simulink model "TosAeb"  (R2021a)
settings: Solver=FixedStepDiscrete  StartTime=0.0  StopTime=inf  FixedStep=0.01  RelTol=1e-3
undefined symbols (not defined in the file or in its folder): ACCModSts, AccSts1, AEB_ObjectMovingOrientation, AEB_ObjectMovingState, CN_BTCActive, CN_CurrentGearR, CN_CurrentGearR_DHT, CN_PTCActive, CN_VDCActive, CN_VSC_EspOff, CN_VSC_FCW_AEB_Error, CN_VSC_FCW_AEB_NoDispay, CN_VSC_FCW_AEB_PerfmDgdtn, CN_VSC_FCW_AEB_TempUnAvlbl, CN_VSC_VehStandstill, CNaccelAvoidMax, CNaccelAvoidMin, CNbrakePedalPress, CNcomfortStrLeftAccelMax, CNcomfortStrLeftAccelMin, CNcomfortStrRightAccelMax, CNcomfortStrRightAccelMin, CNDrvDoorOpen, CNDrvModeEco, CNDrvModeNorm, CNDrvModeOther, CNDrvModeSport, CNDrvSBRreminder, CNNull, CNobjInvalidID, CNPassDoorOpen, CNPassSBRreminder, CNPEBSAccelNegInf, CNPEBSAccelNull, CNPEBSTimeInf, CNPEBSTimeNull, CNSTOPMAX, CNSTOPNULL, CNsubjWidth, CNSwtOFF, CNSwtON, CNTTCMAX, CNTTCNULL, CNVehConfigAEBCycNone, CNVehConfigAEBNone, CNVehConfigAEBPedNone, CNVehConfigFCWNone, CNVehConfigJANone, CollisionSeverity, FALSE, FCWSnvty, JACycObjCalcInfoDefaultParams, JAPedObjCalcInfoDefaultParams, JAVehObjCalcInfoDefaultParams, latCycObjCalcInfoDefaultParams, latPedObjCalcInfoDefaultParams, longObjCalcInfoDefaultParams, ModSts, movingStatus, ObjectType ... (+1112)
libraries: simulink x3031, AEBSFunctionLib x50, Ta_Library x33, AEBSStateMachineLib x23, Mylibrary x12, simulink_extras x7, ObjSelect_lib x3, dspstat3 x2
(part 53 of 102)

== system TosAeb/FcwAebJa_Step_sys/ALG_AEBSystem/ALG_AEBController/AEB_FuncCondCalcApp/LongFunctionCondCalc/vehEBASuppAborCondCalc/vehEBASuppCondCalc/vehEBASituationRelActiveCondCalc/ESPmasterCylindBrakePressDt ==
  ESPmasterCylindBrakePress : Inport
  Constant : Constant  Value=1/Ts
  Gain : Gain  Gain=1/4
  Product : Product
  Subtract : Sum  Inputs=+-
  Sum : Sum  Inputs=+
  Tapped Delay : simulink/Discrete/Tapped Delay  vinit=0.0  samptime=-1  NumDelays=3  DelayOrder=Newest  includeCurrent=on
  Unit Delay : UnitDelay  SampleTime=-1  HasFrameUpgradeWarning=on
  ESPmasterCylindBrakePressDt : Outport
  wires:
  Sum/1 -> Gain/1
  Unit Delay/1 -> Subtract/2
  ESPmasterCylindBrakePress/1 -> Unit Delay/1, Subtract/1
  Subtract/1 -> Product/1
  Constant/1 -> Product/2
  Gain/1 -> ESPmasterCylindBrakePressDt/1
  Product/1 -> Tapped Delay/1
  Tapped Delay/1 -> Sum/1

== system TosAeb/FcwAebJa_Step_sys/ALG_AEBSystem/ALG_AEBController/AEB_FuncCondCalcApp/LongFunctionCondCalc/vehEBASuppAborCondCalc/vehEBASuppCondCalc/vehEBASituationRelActiveCondCalc/brakeReleventSuppCond ==
  VehConfigIbooster : Inport
  predictiveAccelAvoid : Inport  Port=2
  ObjectMovingState : Inport  Port=3
  subjVel : Inport  Port=4
  ESPmasterCylindBrakePress : Inport  Port=5
  BrakePedalStatus_G : Inport  Port=6
  subjLongAccelFromSensor : Inport  Port=7
  subjLongAccel : Inport  Port=8
  IB_BrkPedalAppld : Inport  Port=9
  Bus Creator : BusCreator  Inputs=8
  Bus Selector : BusSelector  OutputSignals=predictiveAccelAvoid,ObjectMovingState,subjVel,ESPmasterCylindBrakePress,BrakePedalStatus_G,subjLongAccelFromSensor,subjLongAccel
  Bus Selector1 : BusSelector  OutputSignals=predictiveAccelAvoid,ObjectMovingState,subjVel,subjLongAccelFromSensor,subjLongAccel,IB_BrkPedalAppld
  Compare To Constant : simulink/Logic and Bit Operations/Compare To Constant  relop===  const=FALSE  OutDataTypeStr=boolean
  If : If  IfExpression=u1
  Merge : Merge
  brakeReleventSuppCond_ESP : Subsystem  VariantControl=(default)
  brakeReleventSuppCond_IBooster : Subsystem  VariantControl=P_AEB_PowerTrainConfig == 1
  brakeReleventSuppCond : Outport
  wires:
  If/2 -> brakeReleventSuppCond_IBooster/Action
  Bus Selector1/1 -> brakeReleventSuppCond_IBooster/1(predictiveAccelAvoid)  "<predictiveAccelAvoid>"
  Bus Selector1/6 -> brakeReleventSuppCond_IBooster/6(IB_BrkPedalAppld)  "<IB_BrkPedalAppld>"
  Bus Selector1/2 -> brakeReleventSuppCond_IBooster/2(ObjectMovingState)  "<ObjectMovingState>"
  Bus Selector1/5 -> brakeReleventSuppCond_IBooster/5(subjLongAccel)  "<subjLongAccel>"
  Bus Selector1/3 -> brakeReleventSuppCond_IBooster/3(subjVel)  "<subjVel>"
  Bus Selector1/4 -> brakeReleventSuppCond_IBooster/4(subjLongAccelFromSensor)  "<subjLongAccelFromSensor>"
  Bus Selector/7 -> brakeReleventSuppCond_ESP/7(subjLongAccel)  "<subjLongAccel>"
  Bus Selector/6 -> brakeReleventSuppCond_ESP/6(subjLongAccelFromSensor)  "<subjLongAccelFromSensor>"
  Bus Selector/5 -> brakeReleventSuppCond_ESP/5(BrakePedalStatus_G)  "<BrakePedalStatus_G>"
  Bus Selector/4 -> brakeReleventSuppCond_ESP/4(ESPmasterCylindBrakePress)  "<ESPmasterCylindBrakePress>"
  Bus Selector/1 -> brakeReleventSuppCond_ESP/1(predictiveAccelAvoid)  "<predictiveAccelAvoid>"
  Bus Selector/3 -> brakeReleventSuppCond_ESP/3(subjVel)  "<subjVel>"
  Bus Selector/2 -> brakeReleventSuppCond_ESP/2(ObjectMovingState)  "<ObjectMovingState>"
  Bus Creator/1 -> Bus Selector/1, Bus Selector1/1
  predictiveAccelAvoid/1 -> Bus Creator/1  "predictiveAccelAvoid"
  ObjectMovingState/1 -> Bus Creator/2  "ObjectMovingState"
  subjVel/1 -> Bus Creator/3  "subjVel"
  ESPmasterCylindBrakePress/1 -> Bus Creator/4  "ESPmasterCylindBrakePress"
  BrakePedalStatus_G/1 -> Bus Creator/5  "BrakePedalStatus_G"
  subjLongAccelFromSensor/1 -> Bus Creator/6  "subjLongAccelFromSensor"
  subjLongAccel/1 -> Bus Creator/7  "subjLongAccel"
  IB_BrkPedalAppld/1 -> Bus Creator/8  "IB_BrkPedalAppld"
  Merge/1 -> brakeReleventSuppCond/1
  brakeReleventSuppCond_IBooster/1(brkTriggerCondFulfilled) -> Merge/2
  brakeReleventSuppCond_ESP/1(brkTriggerCondFulfilled) -> Merge/1
  If/1 -> brakeReleventSuppCond_ESP/Action
  Compare To Constant/1 -> If/1
  VehConfigIbooster/1 -> Compare To Constant/1

== system TosAeb/FcwAebJa_Step_sys/ALG_AEBSystem/ALG_AEBController/AEB_FuncCondCalcApp/LongFunctionCondCalc/vehEBASuppAborCondCalc/vehEBASuppCondCalc/vehEBASituationRelActiveCondCalc/overlapThesCalc ==
  ObjectTypeCameraConfirmed : Inport
  dxFrontBumper : Inport  Port=2
  Compare To Constant : simulink/Logic and Bit Operations/Compare To Constant  relop===  const=TRUE  OutDataTypeStr=boolean
  If : If  IfExpression=u1
  If Action Subsystem : Subsystem  TreatAsAtomicUnit=on
  If Action Subsystem1 : Subsystem  TreatAsAtomicUnit=on
  Merge : Merge
  overlapThes : Outport
  wires:
  ObjectTypeCameraConfirmed/1 -> Compare To Constant/1
  dxFrontBumper/1 -> If Action Subsystem1/1(dxFrontBumper), If Action Subsystem/1(dxFrontBumper)
  If Action Subsystem/1(overlapThes) -> Merge/1
  Compare To Constant/1 -> If/1
  [ObjectNoFusion] -> If Action Subsystem1/Action
  Merge/1 -> overlapThes/1
  If/2 -> [ObjectNoFusion]
  If Action Subsystem1/1(overlapThes) -> Merge/2
  If/1 -> If Action Subsystem/Action

== system TosAeb/FcwAebJa_Step_sys/ALG_AEBSystem/ALG_AEBController/AEB_FuncCondCalcApp/LongFunctionCondCalc/vehEBASuppAborCondCalc/vehEBASuppCondCalc/vehEBASituationRelActiveCondCalc/vehEBASituationRelActiveCondFulfilledCalc ==
  predictiveAccelAvoid : Inport
  PreWarningFuncImpArb : Inport  Port=2
  overlapRatio : Inport  Port=3
  longCollisionSeverity : Inport  Port=4
  overlapThes : Inport  Port=5
  brkTriggerCondFulfilled : Inport  Port=6
  ESPmasterCylindBrakePressDt : Inport  Port=7
  Compare To Constant : simulink/Logic and Bit Operations/Compare To Constant  relop===  const=ModSts.ActiveState  OutDataTypeStr=boolean
  Compare To Constant1 : simulink/Logic and Bit Operations/Compare To Constant  relop===  const=TRUE  OutDataTypeStr=boolean
  Compare To Constant2 : simulink/Logic and Bit Operations/Compare To Constant  relop===  const=CollisionSeverity.High  OutDataTypeStr=boolean
  Compare To Constant3 : simulink/Logic and Bit Operations/Compare To Constant  relop=>=  const=P_AEB_Func_vehEBA_MCBrkPressDtActivationThres  OutDataTypeStr=boolean
  Constant : Constant  Value=P_AEB_Func_vehEBADgrLvlAccelAvoidThresWithWarning
  Constant1 : Constant  Value=P_AEB_Func_vehEBADgrLvlAccelAvoidThresWithoutWarning
  Constant2 : Constant  Value=P_AEB_Func_vehEBA_MCBrkPressDtConfig
  Constant3 : Constant  Value=TRUE
  Logical Operator1 : Logic  Inputs=5  AllPortsSameDT=off  OutDataTypeStr=boolean
  Relational Operator : RelationalOperator  Operator=>  OutDataTypeStr=boolean  RndMeth=Simplest
  Relational Operator1 : RelationalOperator  Operator=<  OutDataTypeStr=boolean  RndMeth=Simplest
  Switch : Switch  Criteria=u2 ~= 0
  Switch1 : Switch  Criteria=u2 ~= 0
  vehEBASituationRelActiveCondFulfilled : Outport
  wires:
  Constant3/1 -> Switch1/3
  Constant2/1 -> Switch1/2
  Switch1/1 -> Logical Operator1/5
  Compare To Constant3/1 -> Switch1/1  "MCBrkPressDt"
  ESPmasterCylindBrakePressDt/1 -> Compare To Constant3/1
  longCollisionSeverity/1 -> Compare To Constant2/1
  Compare To Constant2/1 -> Logical Operator1/4  "longCollisionSeverityCondFulfilled"
  Compare To Constant1/1 -> Logical Operator1/3  "brkTriggerCondFulfilled"
  Relational Operator/1 -> Logical Operator1/2  "OverlapCondFulfilled"
  overlapRatio/1 -> Relational Operator/1
  predictiveAccelAvoid/1 -> Relational Operator1/1
  Switch/1 -> Relational Operator1/2
  Compare To Constant/1 -> Switch/2
  Constant1/1 -> Switch/3
  Constant/1 -> Switch/1
  Logical Operator1/1 -> vehEBASituationRelActiveCondFulfilled/1
  Relational Operator1/1 -> Logical Operator1/1  "collisionLvlCondFulfilled"
  PreWarningFuncImpArb/1 -> Compare To Constant/1
  overlapThes/1 -> Relational Operator/2
  brkTriggerCondFulfilled/1 -> Compare To Constant1/1

== system TosAeb/FcwAebJa_Step_sys/ALG_AEBSystem/ALG_AEBController/AEB_FuncCondCalcApp/LongFunctionCondCalc/vehEBASuppAborCondCalc/vehEBASuppCondCalc/vehEBAVehRelActiveCondCalc/DriverCondJudge ==
  SteerWheelAngle : Inport
  SteerWheelSpd : Inport  Port=2
  AccrPedlPosnDiagc : Inport  Port=3
  Abs : Abs
  Abs1 : Abs
  Compare To Constant : simulink/Logic and Bit Operations/Compare To Constant  relop=>  const=P_AEB_Func_vehEBAStrWheelAngleActivationThres  OutDataTypeStr=boolean
  Compare To Constant1 : simulink/Logic and Bit Operations/Compare To Constant  relop=>  const=P_AEB_Func_vehEBAStrWheelAngleSpdActivationThres  OutDataTypeStr=boolean
  Compare To Constant4 : simulink/Logic and Bit Operations/Compare To Constant  relop=>  const=P_AEB_Func_vehEBAGasPedalActivationThres  OutDataTypeStr=boolean
  Constant : Constant  Value=P_AEB_Func_vehEBAStrCondAborDelayTime
  Constant1 : Constant  Value=Ts
  Constant2 : Constant  Value=P_AEB_Func_vehEBAAccrCondAborDelayTime
  Constant3 : Constant  Value=0.02
  Logical Operator : Logic  Operator=OR  AllPortsSameDT=off  OutDataTypeStr=boolean
  Logical Operator2 : Logic  AllPortsSameDT=off  OutDataTypeStr=boolean
  accrlReleventSuppCondStateDelayAbortion : AEBSFunctionLib/StateDelayAbortion  SystemSampleTime=-1  VariantActivationTime=update diagram  Latency=0  AutoFrameSizeCalculation=off
  strReleventSuppCondStateDelayAbortion : AEBSFunctionLib/StateDelayAbortion  SystemSampleTime=-1  VariantActivationTime=update diagram  Latency=0  AutoFrameSizeCalculation=off
  driverSuppCondFlg : Outport
  wires:
  Constant3/1 -> accrlReleventSuppCondStateDelayAbortion/3
  Constant2/1 -> accrlReleventSuppCondStateDelayAbortion/2
  Compare To Constant4/1 -> accrlReleventSuppCondStateDelayAbortion/1
  Constant1/1 -> strReleventSuppCondStateDelayAbortion/3
  Constant/1 -> strReleventSuppCondStateDelayAbortion/2
  Logical Operator/1 -> strReleventSuppCondStateDelayAbortion/1
  Logical Operator2/1 -> driverSuppCondFlg/1  "driverSuppCondFlg"
  accrlReleventSuppCondStateDelayAbortion/1 -> Logical Operator2/2  "accrlReleventSuppCond"
  AccrPedlPosnDiagc/1 -> Compare To Constant4/1
  strReleventSuppCondStateDelayAbortion/1 -> Logical Operator2/1  "strReleventSuppCond"
  Compare To Constant1/1 -> Logical Operator/2
  Compare To Constant/1 -> Logical Operator/1
  Abs1/1 -> Compare To Constant1/1
  SteerWheelSpd/1 -> Abs1/1
  Abs/1 -> Compare To Constant/1
  SteerWheelAngle/1 -> Abs/1

== system TosAeb/FcwAebJa_Step_sys/ALG_AEBSystem/ALG_AEBController/AEB_FuncCondCalcApp/LongFunctionCondCalc/vehEBASuppAborCondCalc/vehEBASuppCondCalc/vehEBAVehRelActiveCondCalc/subjVelWork ==
  ObjectMovingState : Inport
  Compare To Constant : simulink/Logic and Bit Operations/Compare To Constant  relop===  const=AEB_ObjectMovingState.Still  OutDataTypeStr=boolean
  Constant : Constant  Value=P_AEB_Func_vehEBASubjVelMaxSO
  Constant2 : Constant  Value=P_AEB_Func_vehEBASubjVelMin
  Constant3 : Constant  Value=P_AEB_Func_vehEBASubjVelMaxMO
  Switch1 : Switch  Criteria=u2 ~= 0
  subjVelWorkMax : Outport
  subjVelWorkMin : Outport  Port=2
  wires:
  Constant/1 -> Switch1/1
  ObjectMovingState/1 -> Compare To Constant/1
  Constant2/1 -> subjVelWorkMin/1
  Compare To Constant/1 -> Switch1/2
  Constant3/1 -> Switch1/3
  Switch1/1 -> subjVelWorkMax/1

== system TosAeb/FcwAebJa_Step_sys/ALG_AEBSystem/ALG_AEBController/AEB_FuncCondCalcApp/LongFunctionCondCalc/vehEBASuppAborCondCalc/vehEBASuppCondCalc/vehEBAVehRelActiveCondCalc/vehEBAVehRelActiveCondJudge ==
  subjVel : Inport
  subjVelWorkMax : Inport  Port=2
  subjVelWorkMin : Inport  Port=3
  driverSuppCondFlg : Inport  Port=4
  vehStateCalcEBASuppFlg : Inport  Port=5
  Compare To Constant : simulink/Logic and Bit Operations/Compare To Constant  relop===  const=FALSE  OutDataTypeStr=boolean
  Compare To Constant1 : simulink/Logic and Bit Operations/Compare To Constant  relop===  const=FALSE  OutDataTypeStr=boolean
  Logical Operator : Logic  AllPortsSameDT=off  OutDataTypeStr=boolean
  Logical Operator1 : Logic  Inputs=3  AllPortsSameDT=off  OutDataTypeStr=boolean
  Relational Operator : RelationalOperator  Operator=<  OutDataTypeStr=boolean  RndMeth=Simplest
  Relational Operator1 : RelationalOperator  Operator=>  OutDataTypeStr=boolean  RndMeth=Simplest
  vehEBAVehRelActiveCondFulfilled : Outport
  wires:
  subjVelWorkMin/1 -> Relational Operator1/2
  Logical Operator1/1 -> vehEBAVehRelActiveCondFulfilled/1
  Logical Operator/1 -> Logical Operator1/1
  Compare To Constant/1 -> Logical Operator1/2
  vehStateCalcEBASuppFlg/1 -> Compare To Constant1/1
  driverSuppCondFlg/1 -> Compare To Constant/1
  Relational Operator1/1 -> Logical Operator/2
  Relational Operator/1 -> Logical Operator/1
  subjVelWorkMax/1 -> Relational Operator/2
  subjVel/1 -> Relational Operator/1, Relational Operator1/1
  Compare To Constant1/1 -> Logical Operator1/3

== system TosAeb/FcwAebJa_Step_sys/ALG_AEBSystem/ALG_AEBController/AEB_FuncCondCalcApp/LongFunctionCondCalc/vehLatentWarningSuppAborCondFulfilled/vehLatentWarningAborCondCalc/vehLatentWarningSituationRelAborCondCalc/If Action Subsystem ==
  Action Port : ActionPort  ActionPortLabel=if(u1)
  Constant : Constant  Value=CNNull
  latentWarningTimeGap : Outport
  wires:
  Constant/1 -> latentWarningTimeGap/1

== system TosAeb/FcwAebJa_Step_sys/ALG_AEBSystem/ALG_AEBController/AEB_FuncCondCalcApp/LongFunctionCondCalc/vehLatentWarningSuppAborCondFulfilled/vehLatentWarningAborCondCalc/vehLatentWarningSituationRelAborCondCalc/If Action Subsystem1 ==
  dxFrontBumper : Inport
  subjVel : Inport  Port=2
  Action Port : ActionPort  ActionPortLabel=else
  Divide : Product  Inputs=*/
  latentWarningTimeGap : Outport
  wires:
  Divide/1 -> latentWarningTimeGap/1
  subjVel/1 -> Divide/2
  dxFrontBumper/1 -> Divide/1

== system TosAeb/FcwAebJa_Step_sys/ALG_AEBSystem/ALG_AEBController/AEB_FuncCondCalcApp/LongFunctionCondCalc/vehLatentWarningSuppAborCondFulfilled/vehLatentWarningAborCondCalc/vehLatentWarningSituationRelAborCondCalc/timer ==
  cond : Inport
  If Action Subsystem2 : Subsystem  TreatAsAtomicUnit=on
  If Action Subsystem3 : Subsystem  TreatAsAtomicUnit=on
  If1 : If  IfExpression=u1
  Merge1 : Merge
  time : Outport
  wires:
  cond/1 -> If1/1
  Merge1/1 -> time/1  "time"
  If Action Subsystem3/1(time) -> Merge1/2
  If1/2 -> If Action Subsystem3/Action
  If Action Subsystem2/1(time) -> Merge1/1
  If1/1 -> If Action Subsystem2/Action

== system TosAeb/FcwAebJa_Step_sys/ALG_AEBSystem/ALG_AEBController/AEB_FuncCondCalcApp/LongFunctionCondCalc/vehLatentWarningSuppAborCondFulfilled/vehLatentWarningAborCondCalc/vehLatentWarningVehRelActiveCondCalc/ACCStateJudge ==
  ACCModSts : Inport
  Compare To Constant : simulink/Logic and Bit Operations/Compare To Constant  relop===  const=ACCModSts.ActiveControlMode  OutDataTypeStr=boolean
  Compare To Constant1 : simulink/Logic and Bit Operations/Compare To Constant  relop===  const=ACCModSts.OverrideMode  OutDataTypeStr=boolean
  Logical Operator : Logic  Operator=OR  AllPortsSameDT=off  OutDataTypeStr=boolean
  ACCStateSuppCondFlg : Outport
  wires:
  Logical Operator/1 -> ACCStateSuppCondFlg/1
  Compare To Constant1/1 -> Logical Operator/2
  Compare To Constant/1 -> Logical Operator/1
  ACCModSts/1 -> Compare To Constant/1, Compare To Constant1/1

== system TosAeb/FcwAebJa_Step_sys/ALG_AEBSystem/ALG_AEBController/AEB_FuncCondCalcApp/LongFunctionCondCalc/vehLatentWarningSuppAborCondFulfilled/vehLatentWarningAborCondCalc/vehLatentWarningVehRelActiveCondCalc/vehLatentWarningVehRelAborCondJudge ==
  vehStateCalcLatentWarningAborFlg : Inport
  subjVel : Inport  Port=2
  ACCStateSuppCondFlg : Inport  Port=3
  Compare To Constant : simulink/Logic and Bit Operations/Compare To Constant  relop===  const=TRUE  OutDataTypeStr=boolean
  Compare To Constant1 : simulink/Logic and Bit Operations/Compare To Constant  relop===  const=TRUE  OutDataTypeStr=boolean
  Compare To Constant2 : simulink/Logic and Bit Operations/Compare To Constant  relop=<=  const=P_AEB_Func_vehLatentWarningSubjVelAborThres  OutDataTypeStr=boolean
  Logical Operator1 : Logic  Operator=OR  Inputs=3  AllPortsSameDT=off  OutDataTypeStr=boolean
  vehLatentWarningVehRelAborCondFulfilled : Outport
  wires:
  Compare To Constant/1 -> Logical Operator1/3
  Compare To Constant2/1 -> Logical Operator1/2
  Compare To Constant1/1 -> Logical Operator1/1
  subjVel/1 -> Compare To Constant2/1
  Logical Operator1/1 -> vehLatentWarningVehRelAborCondFulfilled/1
  vehStateCalcLatentWarningAborFlg/1 -> Compare To Constant1/1
  ACCStateSuppCondFlg/1 -> Compare To Constant/1

== system TosAeb/FcwAebJa_Step_sys/ALG_AEBSystem/ALG_AEBController/AEB_FuncCondCalcApp/LongFunctionCondCalc/vehLatentWarningSuppAborCondFulfilled/vehLatentWarningSuppCondCalc/vehLatentWarningSituationRelActiveCondCalc/If Action Subsystem ==
  Action Port : ActionPort  ActionPortLabel=if(u1)
  Constant : Constant  Value=CNNull
  latentWarningTimeGap : Outport
  wires:
  Constant/1 -> latentWarningTimeGap/1

== system TosAeb/FcwAebJa_Step_sys/ALG_AEBSystem/ALG_AEBController/AEB_FuncCondCalcApp/LongFunctionCondCalc/vehLatentWarningSuppAborCondFulfilled/vehLatentWarningSuppCondCalc/vehLatentWarningSituationRelActiveCondCalc/If Action Subsystem1 ==
  dxFrontBumper : Inport
  subjVel : Inport  Port=2
  Action Port : ActionPort  ActionPortLabel=else
  Divide : Product  Inputs=*/
  latentWarningTimeGap : Outport
  wires:
  Divide/1 -> latentWarningTimeGap/1
  subjVel/1 -> Divide/2
  dxFrontBumper/1 -> Divide/1

== system TosAeb/FcwAebJa_Step_sys/ALG_AEBSystem/ALG_AEBController/AEB_FuncCondCalcApp/LongFunctionCondCalc/vehLatentWarningSuppAborCondFulfilled/vehLatentWarningSuppCondCalc/vehLatentWarningSituationRelActiveCondCalc/TimeThesCalc ==
  FCWSnvty : Inport
  Bus Creator{,1..3} (4 alike) : BusCreator  Inputs=2
  Bus Selector : BusSelector  OutputSignals=timeGapThes,prolongTime
  Multiport Switch : MultiPortSwitch  DataPortOrder=Specify indices  DataPortIndices={FCWSnvty.HMISettingEarly,FCWSnvty.HMISettingMiddle,FCWSnvty.HMISettingLate}  DataPortForDefault=Additional data port
  ProlongTimeDefault : Constant  Value=P_AEB_Func_vehLatentWarningTimeGapDurationThresDefault
  ProlongTimeHMISettingEarly : Constant  Value=P_AEB_Func_vehLatentWarningTimeGapDurationThresEarly
  ProlongTimeHMISettingLate : Constant  Value=P_AEB_Func_vehLatentWarningTimeGapDurationThresLate
  ProlongTimeHMISettingMiddle : Constant  Value=P_AEB_Func_vehLatentWarningTimeGapDurationThresMiddle
  TimeGapThesDefault : Constant  Value=P_AEB_Func_vehLatentWarningTimeGapThresDefault
  TimeGapThesHMISettingEarly : Constant  Value=P_AEB_Func_vehLatentWarningTimeGapThresHmiEarly
  TimeGapThesHMISettingLate : Constant  Value=P_AEB_Func_vehLatentWarningTimeGapThresHmiLate
  TimeGapThesHMISettingMiddle : Constant  Value=P_AEB_Func_vehLatentWarningTimeGapThresHmiMiddle
  timeGapThes : Outport
  prolongTime : Outport  Port=2
  wires:
  Bus Selector/2 -> prolongTime/1  "<prolongTime>"
  Bus Selector/1 -> timeGapThes/1  "<timeGapThes>"
  Multiport Switch/1 -> Bus Selector/1
  TimeGapThesDefault/1 -> Bus Creator3/1  "timeGapThes"
  ProlongTimeDefault/1 -> Bus Creator3/2  "prolongTime"
  ProlongTimeHMISettingEarly/1 -> Bus Creator/2  "prolongTime"
  TimeGapThesHMISettingLate/1 -> Bus Creator2/1  "timeGapThes"
  ProlongTimeHMISettingMiddle/1 -> Bus Creator1/2  "prolongTime"
  ProlongTimeHMISettingLate/1 -> Bus Creator2/2  "prolongTime"
  TimeGapThesHMISettingMiddle/1 -> Bus Creator1/1  "timeGapThes"
  Bus Creator/1 -> Multiport Switch/2
  FCWSnvty/1 -> Multiport Switch/1
  Bus Creator2/1 -> Multiport Switch/4
  Bus Creator1/1 -> Multiport Switch/3
  Bus Creator3/1 -> Multiport Switch/5
  TimeGapThesHMISettingEarly/1 -> Bus Creator/1  "timeGapThes"

== system TosAeb/FcwAebJa_Step_sys/ALG_AEBSystem/ALG_AEBController/AEB_FuncCondCalcApp/LongFunctionCondCalc/vehLatentWarningSuppAborCondFulfilled/vehLatentWarningSuppCondCalc/vehLatentWarningVehRelActiveCondCalc/ACCStateJudge ==
  ACCModSts : Inport
  Compare To Constant : simulink/Logic and Bit Operations/Compare To Constant  relop===  const=ACCModSts.ActiveControlMode  OutDataTypeStr=boolean
  Compare To Constant1 : simulink/Logic and Bit Operations/Compare To Constant  relop===  const=ACCModSts.OverrideMode  OutDataTypeStr=boolean
  Logical Operator : Logic  Operator=OR  AllPortsSameDT=off  OutDataTypeStr=boolean
  ACCStateSuppCondFlg : Outport
  wires:
  Logical Operator/1 -> ACCStateSuppCondFlg/1
  Compare To Constant1/1 -> Logical Operator/2
  Compare To Constant/1 -> Logical Operator/1
  ACCModSts/1 -> Compare To Constant1/1, Compare To Constant/1

== system TosAeb/FcwAebJa_Step_sys/ALG_AEBSystem/ALG_AEBController/AEB_FuncCondCalcApp/LongFunctionCondCalc/vehLatentWarningSuppAborCondFulfilled/vehLatentWarningSuppCondCalc/vehLatentWarningVehRelActiveCondCalc/subjVelWork ==
  Constant1 : Constant  Value=P_AEB_Func_vehLatentWarningSubjVelMax
  Constant2 : Constant  Value=P_AEB_Func_vehLatentWarningSubjVelMin
  subjVelWorkMax : Outport
  subjVelWorkMin : Outport  Port=2
  wires:
  Constant1/1 -> subjVelWorkMax/1
  Constant2/1 -> subjVelWorkMin/1

== system TosAeb/FcwAebJa_Step_sys/ALG_AEBSystem/ALG_AEBController/AEB_FuncCondCalcApp/LongFunctionCondCalc/vehLatentWarningSuppAborCondFulfilled/vehLatentWarningSuppCondCalc/vehLatentWarningVehRelActiveCondCalc/vehLatentWarningVehRelActiveCondJudge ==
  subjVel : Inport
  subjVelWorkMax : Inport  Port=2
  subjVelWorkMin : Inport  Port=3
  ACCStateSuppCondFlg : Inport  Port=4
  vehStateCalcLatentWarningSuppFlg : Inport  Port=5
  Compare To Constant : simulink/Logic and Bit Operations/Compare To Constant  relop===  const=FALSE  OutDataTypeStr=boolean
  Compare To Constant1 : simulink/Logic and Bit Operations/Compare To Constant  relop===  const=FALSE  OutDataTypeStr=boolean
  Logical Operator : Logic  AllPortsSameDT=off  OutDataTypeStr=boolean
  Logical Operator1 : Logic  Inputs=3  AllPortsSameDT=off  OutDataTypeStr=boolean
  Relational Operator : RelationalOperator  Operator=<  OutDataTypeStr=boolean  RndMeth=Simplest
  Relational Operator1 : RelationalOperator  Operator=>  OutDataTypeStr=boolean  RndMeth=Simplest
  vehLatentWarningVehRelActiveCondFulfilled : Outport
  wires:
  subjVel/1 -> Relational Operator1/1, Relational Operator/1
  subjVelWorkMax/1 -> Relational Operator/2
  subjVelWorkMin/1 -> Relational Operator1/2
  Relational Operator/1 -> Logical Operator/1
  Relational Operator1/1 -> Logical Operator/2
  ACCStateSuppCondFlg/1 -> Compare To Constant/1
  vehStateCalcLatentWarningSuppFlg/1 -> Compare To Constant1/1
  Compare To Constant/1 -> Logical Operator1/2
  Compare To Constant1/1 -> Logical Operator1/3
  Logical Operator/1 -> Logical Operator1/1
  Logical Operator1/1 -> vehLatentWarningVehRelActiveCondFulfilled/1

== system TosAeb/FcwAebJa_Step_sys/ALG_AEBSystem/ALG_AEBController/AEB_FuncCondCalcApp/LongFunctionCondCalc/vehPreWarningSuppAborCondFulfilled/vehPreWarningAborCondCalc/vehPreWarningVehRelActiveCondCalc/DriverCondJudge ==
  SteerWheelAngle : Inport
  SteerWheelSpd : Inport  Port=2
  AccrPedlPosnDiagc : Inport  Port=3
  Abs : Abs
  Abs1 : Abs
  Compare To Constant : simulink/Logic and Bit Operations/Compare To Constant  relop=>  const=P_AEB_Func_vehPreWarningStrWheelAngleAborThres  OutDataTypeStr=boolean
  Compare To Constant1 : simulink/Logic and Bit Operations/Compare To Constant  relop=>  const=P_AEB_Func_vehPreWarningStrWheelAngleSpdAborThres  OutDataTypeStr=boolean
  Compare To Constant4 : simulink/Logic and Bit Operations/Compare To Constant  relop=>  const=P_AEB_Func_vehPreWarningGasPedalAborThres  OutDataTypeStr=boolean
  Logical Operator : Logic  Operator=OR  AllPortsSameDT=off  OutDataTypeStr=boolean
  Logical Operator2 : Logic  Operator=OR  AllPortsSameDT=off  OutDataTypeStr=boolean
  driverAborCondFlg : Outport
  wires:
  SteerWheelAngle/1 -> Abs/1
  Abs/1 -> Compare To Constant/1
  SteerWheelSpd/1 -> Abs1/1
  Abs1/1 -> Compare To Constant1/1
  Compare To Constant/1 -> Logical Operator/1
  Compare To Constant1/1 -> Logical Operator/2
  Logical Operator/1 -> Logical Operator2/1  "strReleventAborCond"
  AccrPedlPosnDiagc/1 -> Compare To Constant4/1
  Compare To Constant4/1 -> Logical Operator2/2  "accrlReleventAborCond"
  Logical Operator2/1 -> driverAborCondFlg/1  "driverAborCond"

== system TosAeb/FcwAebJa_Step_sys/ALG_AEBSystem/ALG_AEBController/AEB_FuncCondCalcApp/LongFunctionCondCalc/vehPreWarningSuppAborCondFulfilled/vehPreWarningAborCondCalc/vehPreWarningVehRelActiveCondCalc/vehPreWarningVehRelAborCondJudge ==
  driverAborCondFlg : Inport
  vehStateCalcPreWarningAborFlg : Inport  Port=2
  EBAFuncImpArb : Inport  Port=3
  Compare To Constant : simulink/Logic and Bit Operations/Compare To Constant  relop===  const=TRUE  OutDataTypeStr=boolean
  Compare To Constant1 : simulink/Logic and Bit Operations/Compare To Constant  relop===  const=TRUE  OutDataTypeStr=boolean
  Compare To Constant2 : simulink/Logic and Bit Operations/Compare To Constant  relop===  const=ModSts.ActiveState  OutDataTypeStr=boolean
  Logical Operator1 : Logic  Inputs=3  AllPortsSameDT=off  OutDataTypeStr=boolean
  vehPreWarningVehRelAborCondFulfilled : Outport
  wires:
  Compare To Constant2/1 -> Logical Operator1/3
  Compare To Constant1/1 -> Logical Operator1/2
  Compare To Constant/1 -> Logical Operator1/1
  EBAFuncImpArb/1 -> Compare To Constant2/1
  driverAborCondFlg/1 -> Compare To Constant/1
  vehStateCalcPreWarningAborFlg/1 -> Compare To Constant1/1
  Logical Operator1/1 -> vehPreWarningVehRelAborCondFulfilled/1  "vehPreWarningVehRelAborCondFulfilled"

== system TosAeb/FcwAebJa_Step_sys/ALG_AEBSystem/ALG_AEBController/AEB_FuncCondCalcApp/LongFunctionCondCalc/vehPreWarningSuppAborCondFulfilled/vehPreWarningSuppCondCalc/vehPreWarningSituationRelActiveCondCalc/IsTtcCondFulfilledCalc ==
  TTC : Inport
  FcwHMISetting : Inport  Port=2
  subjVel : Inport  Port=3  OutDataTypeStr=single
  ACCModSts : Inport  Port=4
  Add : Sum  Inputs=+-
  Compare To Constant : simulink/Logic and Bit Operations/Compare To Constant  relop===  const=ACCModSts.ActiveControlMode  OutDataTypeStr=boolean
  Constant : Constant  Value=P_AEB_Func_VehPreWarningTTC_OffsetOfACC
  LowLvlBrkAccelThesCalcHMIEarly : Subsystem  TreatAsAtomicUnit=on
  LowLvlBrkAccelThesCalcHMILate : Subsystem  TreatAsAtomicUnit=on
  LowLvlBrkAccelThesCalcHMIMid : Subsystem  TreatAsAtomicUnit=on
  Merge : Merge  Inputs=3
  Relational Operator1 : RelationalOperator  Operator=<=  OutDataTypeStr=boolean  RndMeth=Simplest
  Switch : Switch  Criteria=u2 ~= 0
  Switch Case : SwitchCase  CaseConditions={FCWSnvty.HMISettingEarly,FCWSnvty.HMISettingLate}
  IsTtcCondFulfilled : Outport
  wires:
  Switch Case/3 -> [DEFAULT]
  TTC/1 -> Relational Operator1/1
  [HMIEarly] -> LowLvlBrkAccelThesCalcHMIEarly/Action
  Switch Case/1 -> [HMIEarly]
  Switch Case/2 -> [HMILate]
  FcwHMISetting/1 -> Switch Case/1
  LowLvlBrkAccelThesCalcHMILate/1(VehPreWarning_TTC_Thres) -> Merge/2
  [DEFAULT] -> LowLvlBrkAccelThesCalcHMIMid/Action
  subjVel/1 -> LowLvlBrkAccelThesCalcHMIEarly/1(subjVel), LowLvlBrkAccelThesCalcHMIMid/1(subjVel), LowLvlBrkAccelThesCalcHMILate/1(subjVel)
  Merge/1 -> Add/1, Switch/3  "AEB_Func_VehPreWarning_TTC_Thres"
  LowLvlBrkAccelThesCalcHMIMid/1(VehPreWarning_TTC_Thres) -> Merge/3
  [HMILate] -> LowLvlBrkAccelThesCalcHMILate/Action
  Relational Operator1/1 -> IsTtcCondFulfilled/1
  LowLvlBrkAccelThesCalcHMIEarly/1(VehPreWarning_TTC_Thres) -> Merge/1
  ACCModSts/1 -> Compare To Constant/1
  Compare To Constant/1 -> Switch/2
  Constant/1 -> Add/2
  Add/1 -> Switch/1
  Switch/1 -> Relational Operator1/2

== system TosAeb/FcwAebJa_Step_sys/ALG_AEBSystem/ALG_AEBController/AEB_FuncCondCalcApp/LongFunctionCondCalc/vehPreWarningSuppAborCondFulfilled/vehPreWarningSuppCondCalc/vehPreWarningSituationRelActiveCondCalc/Subsystem1 ==
  longCollisionSeverity : Inport  OutDataTypeStr=Enum: CollisionSeverity
  overlapRatio : Inport  Port=2
  dxFrontBumper : Inport  Port=3
  probEscape : Inport  Port=4
  subjCurvTraj : Inport  Port=5
  IsTtcCondFulfilled : Inport  Port=6
  Compare To Constant : simulink/Logic and Bit Operations/Compare To Constant  relop===  const=CollisionSeverity.Low  OutDataTypeStr=boolean
  Compare To Constant1 : simulink/Logic and Bit Operations/Compare To Constant  relop===  const=CollisionSeverity.Medium  OutDataTypeStr=boolean
  Compare To Constant2 : simulink/Logic and Bit Operations/Compare To Constant  relop===  const=CollisionSeverity.High  OutDataTypeStr=boolean
  Compare To Constant3 : simulink/Logic and Bit Operations/Compare To Constant  relop=>  const=P_AEB_Func_vehPreWarningEscapeActivationThres  OutDataTypeStr=boolean
  Compare To Constant4 : simulink/Logic and Bit Operations/Compare To Constant  relop=<  const=P_AEB_Func_vehPreWarningSubjCurvTrajActivationThres  OutDataTypeStr=boolean
  Compare To Constant5 : simulink/Logic and Bit Operations/Compare To Constant  relop===  const=FALSE  OutDataTypeStr=boolean
  Logical Operator : Logic  Operator=OR  Inputs=3  AllPortsSameDT=off  OutDataTypeStr=boolean
  Logical Operator1 : Logic  Inputs=5  AllPortsSameDT=off  OutDataTypeStr=boolean
  OverlapThesLookupTable : Lookup_n-D  NumberOfTableDimensions=1  BreakpointsForDimension1=P_AEB_Func_vehPreWarningOverlapActivationThresX  BeginIndexSearchUsingPreviousIndexResult=on  Table=P_AEB_Func_vehPreWarningOverlapActivationThresY  ExtrapMethod=Clip  InternalRulePriority=Speed  RndMeth=Simplest  InputSameDT=on
  Relational Operator : RelationalOperator  Operator=>  OutDataTypeStr=boolean  RndMeth=Simplest
  vehPreWarningSituationRelActiveCondFulfilled : Outport
  wires:
  OverlapThesLookupTable/1 -> Relational Operator/2
  overlapRatio/1 -> Relational Operator/1
  Compare To Constant3/1 -> Compare To Constant5/1  "probEscapeFulfilled"
  Logical Operator1/1 -> vehPreWarningSituationRelActiveCondFulfilled/1
  Relational Operator/1 -> Logical Operator1/2  "OverlapCondFulfilled"
  dxFrontBumper/1 -> OverlapThesLookupTable/1
  probEscape/1 -> Compare To Constant3/1
  Compare To Constant4/1 -> Logical Operator1/4  "latStableCondFulfilled"
  IsTtcCondFulfilled/1 -> Logical Operator1/5
  Compare To Constant/1 -> Logical Operator/1
  subjCurvTraj/1 -> Compare To Constant4/1
  Compare To Constant2/1 -> Logical Operator/3
  Compare To Constant1/1 -> Logical Operator/2
  longCollisionSeverity/1 -> Compare To Constant2/1, Compare To Constant1/1, Compare To Constant/1
  Logical Operator/1 -> Logical Operator1/1  "collisionLvlCondFulfilled"
  Compare To Constant5/1 -> Logical Operator1/3  "probEscapeFulfilled"

== system TosAeb/FcwAebJa_Step_sys/ALG_AEBSystem/ALG_AEBController/AEB_FuncCondCalcApp/LongFunctionCondCalc/vehPreWarningSuppAborCondFulfilled/vehPreWarningSuppCondCalc/vehPreWarningVehRelActiveCondCalc/DriverCondJudge ==
  SteerWheelAngle : Inport
  SteerWheelSpd : Inport  Port=2
  VehConfigIbooster : Inport  Port=3
  ESPmasterCylindBrakePress : Inport  Port=4
  BrakePedalStatus_G : Inport  Port=5
  IB_BrkPedalAppld : Inport  Port=6
  AccrPedlPosnDiagc : Inport  Port=7
  Abs : Abs
  Abs1 : Abs
  AccrReleventSuppCondStateDelayAbortion : AEBSFunctionLib/StateDelayAbortion  SystemSampleTime=-1  VariantActivationTime=update diagram  Latency=0  AutoFrameSizeCalculation=off
  Compare To Constant : simulink/Logic and Bit Operations/Compare To Constant  relop=>  const=P_AEB_Func_vehPreWarningStrWheelAngleActivationThres  OutDataTypeStr=boolean
  Compare To Constant1 : simulink/Logic and Bit Operations/Compare To Constant  relop=>  const=P_AEB_Func_vehPreWarningStrWheelAngleSpdActivationThres  OutDataTypeStr=boolean
  Compare To Constant4 : simulink/Logic and Bit Operations/Compare To Constant  relop=>  const=P_AEB_Func_vehPreWarningGasPedalActivationThres  OutDataTypeStr=boolean
  Constant : Constant  Value=P_AEB_Func_vehPreWarningStrCondAborDelayTime
  Constant1 : Constant  Value=Ts
  Constant2 : Constant  Value=Ts
  Constant3 : Constant  Value=P_AEB_Func_vehPreWarningAccrCondAborDelayTime
  Logical Operator : Logic  Operator=OR  AllPortsSameDT=off  OutDataTypeStr=boolean
  Logical Operator2 : Logic  Operator=OR  Inputs=3  AllPortsSameDT=off  OutDataTypeStr=boolean
  StrReleventSuppCondDelayAbortion : AEBSFunctionLib/StateDelayAbortion  SystemSampleTime=-1  VariantActivationTime=update diagram  Latency=0  AutoFrameSizeCalculation=off
  brakeReleventSuppCond : Subsystem
  driverSuppCondFlg : Outport
  wires:
  VehConfigIbooster/1 -> brakeReleventSuppCond/1(VehConfigIbooster)
  IB_BrkPedalAppld/1 -> brakeReleventSuppCond/4(IB_BrkPedalAppld)
  brakeReleventSuppCond/1(brakeReleventSuppCond) -> Logical Operator2/2  "brakeReleventSuppCond"
  BrakePedalStatus_G/1 -> brakeReleventSuppCond/3(BrakePedalStatus_G)
  ESPmasterCylindBrakePress/1 -> brakeReleventSuppCond/2(ESPmasterCylindBrakePress)
  Constant3/1 -> AccrReleventSuppCondStateDelayAbortion/2
  Constant2/1 -> AccrReleventSuppCondStateDelayAbortion/3
  Compare To Constant4/1 -> AccrReleventSuppCondStateDelayAbortion/1
  Constant1/1 -> StrReleventSuppCondDelayAbortion/3
  Constant/1 -> StrReleventSuppCondDelayAbortion/2
  Logical Operator2/1 -> driverSuppCondFlg/1  "driverSuppCond"
  AccrReleventSuppCondStateDelayAbortion/1 -> Logical Operator2/3  "accrReleventSuppCond"
  AccrPedlPosnDiagc/1 -> Compare To Constant4/1
  StrReleventSuppCondDelayAbortion/1 -> Logical Operator2/1  "strReleventSuppCond"
  Logical Operator/1 -> StrReleventSuppCondDelayAbortion/1
  Compare To Constant1/1 -> Logical Operator/2
  Compare To Constant/1 -> Logical Operator/1
  Abs1/1 -> Compare To Constant1/1
  SteerWheelSpd/1 -> Abs1/1
  Abs/1 -> Compare To Constant/1
  SteerWheelAngle/1 -> Abs/1

== system TosAeb/FcwAebJa_Step_sys/ALG_AEBSystem/ALG_AEBController/AEB_FuncCondCalcApp/LongFunctionCondCalc/vehPreWarningSuppAborCondFulfilled/vehPreWarningSuppCondCalc/vehPreWarningVehRelActiveCondCalc/subjVelWork ==
  ObjectMovingState : Inport
  Compare To Constant : simulink/Logic and Bit Operations/Compare To Constant  relop===  const=AEB_ObjectMovingState.Still  OutDataTypeStr=boolean
  Constant : Constant  Value=P_AEB_Func_vehPreWarningSubjVelMaxActivationThresSO
  Constant1 : Constant  Value=P_AEB_Func_vehPreWarningSubjVelMaxActivationThresMO
  Constant2 : Constant  Value=P_AEB_Func_vehPreWarningSubjVelMinActivationThres
  Switch : Switch  Criteria=u2 ~= 0
  subjVelWorkMax : Outport
  subjVelWorkMin : Outport  Port=2
  wires:
  Compare To Constant/1 -> Switch/2
  ObjectMovingState/1 -> Compare To Constant/1
  Constant/1 -> Switch/1
  Constant1/1 -> Switch/3
  Switch/1 -> subjVelWorkMax/1
  Constant2/1 -> subjVelWorkMin/1

== system TosAeb/FcwAebJa_Step_sys/ALG_AEBSystem/ALG_AEBController/AEB_FuncCondCalcApp/LongFunctionCondCalc/vehPreWarningSuppAborCondFulfilled/vehPreWarningSuppCondCalc/vehPreWarningVehRelActiveCondCalc/vehPreWarningVehRelActiveCondJudge ==
  subjVel : Inport
  subjVelWorkMax : Inport  Port=2
  subjVelWorkMin : Inport  Port=3
  driverSuppCondFlg : Inport  Port=4
  vehStateCalcPreWarningSuppFlg : Inport  Port=5
  Compare To Constant : simulink/Logic and Bit Operations/Compare To Constant  relop===  const=FALSE  OutDataTypeStr=boolean
  Compare To Constant1 : simulink/Logic and Bit Operations/Compare To Constant  relop===  const=FALSE  OutDataTypeStr=boolean
  Logical Operator : Logic  AllPortsSameDT=off  OutDataTypeStr=boolean
  Logical Operator1 : Logic  Inputs=3  AllPortsSameDT=off  OutDataTypeStr=boolean
  Relational Operator : RelationalOperator  Operator=<  OutDataTypeStr=boolean  RndMeth=Simplest
  Relational Operator1 : RelationalOperator  Operator=>  OutDataTypeStr=boolean  RndMeth=Simplest
  vehPreWarningVehRelActiveCondFulfilled : Outport
  wires:
  Logical Operator1/1 -> vehPreWarningVehRelActiveCondFulfilled/1  "vehPreWarningVehRelActiveCondFulfilled"
  Logical Operator/1 -> Logical Operator1/1
  Compare To Constant1/1 -> Logical Operator1/3
  Compare To Constant/1 -> Logical Operator1/2
  vehStateCalcPreWarningSuppFlg/1 -> Compare To Constant1/1
  driverSuppCondFlg/1 -> Compare To Constant/1
  Relational Operator1/1 -> Logical Operator/2
  Relational Operator/1 -> Logical Operator/1
  subjVelWorkMin/1 -> Relational Operator1/2
  subjVelWorkMax/1 -> Relational Operator/2
  subjVel/1 -> Relational Operator/1, Relational Operator1/1

== system TosAeb/FcwAebJa_Step_sys/ALG_AEBSystem/ALG_AEBController/AEB_FuncCondCalcApp/LongFunctionCondCalc/vehPrefillSuppAborCondCalc/vehPrefillAborCondCalc/vehPrefillVehRelAborCondCalc/DriverAborCondJudge ==
  SteerWheelAngle : Inport
  SteerWheelSpd : Inport  Port=2
  ESPmasterCylindBrakePress : Inport  Port=3
  BrakePedalStatus_G : Inport  Port=4
  AccrPedlPosnDiagc : Inport  Port=5
  Abs : Abs
  Abs1 : Abs
  Compare To Constant : simulink/Logic and Bit Operations/Compare To Constant  relop=>  const=P_AEB_Func_vehPrefillStrWheelAngleAborThres  OutDataTypeStr=boolean
  Compare To Constant1 : simulink/Logic and Bit Operations/Compare To Constant  relop=>  const=P_AEB_Func_vehPrefillStrWheelAngleSpdAborThres  OutDataTypeStr=boolean
  Compare To Constant2 : simulink/Logic and Bit Operations/Compare To Constant  relop=>  const=P_AEB_Func_vehPrefillMasterCylindBrkPressAborThres  OutDataTypeStr=boolean
  Compare To Constant3 : simulink/Logic and Bit Operations/Compare To Constant  relop===  const=CNbrakePedalPress  OutDataTypeStr=boolean
  Compare To Constant4 : simulink/Logic and Bit Operations/Compare To Constant  relop=>  const=P_AEB_Func_vehPrefillGasPedalAborThres  OutDataTypeStr=boolean
  Logical Operator : Logic  Operator=OR  AllPortsSameDT=off  OutDataTypeStr=boolean
  Logical Operator1 : Logic  Operator=OR  AllPortsSameDT=off  OutDataTypeStr=boolean
  Logical Operator2 : Logic  Operator=OR  Inputs=3  AllPortsSameDT=off  OutDataTypeStr=boolean
  driverAborCondFlg : Outport
  wires:
  Compare To Constant2/1 -> Logical Operator1/1
  BrakePedalStatus_G/1 -> Compare To Constant3/1
  Compare To Constant3/1 -> Logical Operator1/2
  Logical Operator1/1 -> Logical Operator2/2  "brakeReleventSuppCond"
  ESPmasterCylindBrakePress/1 -> Compare To Constant2/1
  SteerWheelAngle/1 -> Abs/1
  Abs/1 -> Compare To Constant/1
  SteerWheelSpd/1 -> Abs1/1
  Abs1/1 -> Compare To Constant1/1
  Compare To Constant/1 -> Logical Operator/1
  Compare To Constant1/1 -> Logical Operator/2
  Logical Operator/1 -> Logical Operator2/1  "strReleventAborCond"
  AccrPedlPosnDiagc/1 -> Compare To Constant4/1
  Compare To Constant4/1 -> Logical Operator2/3  "accrlReleventAborCond"
  Logical Operator2/1 -> driverAborCondFlg/1  "driverAborCondFlg"

== system TosAeb/FcwAebJa_Step_sys/ALG_AEBSystem/ALG_AEBController/AEB_FuncCondCalcApp/LongFunctionCondCalc/vehPrefillSuppAborCondCalc/vehPrefillAborCondCalc/vehPrefillVehRelAborCondCalc/vehPreWarningVehRelActiveCondJudge ==
  driverAborCondFlg : Inport
  vehStateCalcPrefillAborFlg : Inport  Port=2
  Compare To Constant : simulink/Logic and Bit Operations/Compare To Constant  relop===  const=TRUE  OutDataTypeStr=boolean
  Compare To Constant1 : simulink/Logic and Bit Operations/Compare To Constant  relop===  const=TRUE  OutDataTypeStr=boolean
  Logical Operator1 : Logic  Operator=OR  AllPortsSameDT=off  OutDataTypeStr=boolean
  vehPrefillVehRelAborCondFulfilled : Outport
  wires:
  driverAborCondFlg/1 -> Compare To Constant/1  "driverAborCondFlg"
  vehStateCalcPrefillAborFlg/1 -> Compare To Constant1/1
  Compare To Constant/1 -> Logical Operator1/1
  Logical Operator1/1 -> vehPrefillVehRelAborCondFulfilled/1  "vehPrefillVehRelAborCondFulfilled"
  Compare To Constant1/1 -> Logical Operator1/2

== system TosAeb/FcwAebJa_Step_sys/ALG_AEBSystem/ALG_AEBController/AEB_FuncCondCalcApp/LongFunctionCondCalc/vehPrefillSuppAborCondCalc/vehPrefillSuppCondCalc/vehPrefillVehRelActiveCondCalc/DriverCondJudge ==
  SteerWheelAngle : Inport
  SteerWheelSpd : Inport  Port=2
  VehConfigIbooster : Inport  Port=3
  ESPmasterCylindBrakePress : Inport  Port=4
  BrakePedalStatus_G : Inport  Port=5
  IB_BrkPedalAppld : Inport  Port=6
  AccrPedlPosnDiagc : Inport  Port=7
  Abs : Abs
  Abs1 : Abs
  Compare To Constant : simulink/Logic and Bit Operations/Compare To Constant  relop=>  const=P_AEB_Func_vehPrefillStrWheelAngleActivationThres  OutDataTypeStr=boolean
  Compare To Constant1 : simulink/Logic and Bit Operations/Compare To Constant  relop=>  const=P_AEB_Func_vehPrefillStrWheelAngleSpdActivationThres  OutDataTypeStr=boolean
  Compare To Constant4 : simulink/Logic and Bit Operations/Compare To Constant  relop=>  const=P_AEB_Func_vehPrefillGasPedalActivationThres  OutDataTypeStr=boolean
  Constant : Constant  Value=P_AEB_Func_vehPrefillStrCondAborDelayTime
  Constant1 : Constant  Value=Ts
  Constant2 : Constant  Value=Ts
  Constant3 : Constant  Value=P_AEB_Func_vehPrefillAccrCondAborDelayTime
  Logical Operator : Logic  Operator=OR  AllPortsSameDT=off  OutDataTypeStr=boolean
  Logical Operator2 : Logic  Operator=OR  Inputs=3  AllPortsSameDT=off  OutDataTypeStr=boolean
  accrlReleventSuppCondStateDelayAbortion : AEBSFunctionLib/StateDelayAbortion  SystemSampleTime=-1  VariantActivationTime=update diagram  Latency=0  AutoFrameSizeCalculation=off
  brakeReleventSuppCond : Subsystem
  strReleventSuppCondStateDelayAbortion : AEBSFunctionLib/StateDelayAbortion  SystemSampleTime=-1  VariantActivationTime=update diagram  Latency=0  AutoFrameSizeCalculation=off
  driverSuppCondFlg : Outport
  wires:
  VehConfigIbooster/1 -> brakeReleventSuppCond/1(VehConfigIbooster)
  IB_BrkPedalAppld/1 -> brakeReleventSuppCond/4(IB_BrkPedalAppld)
  BrakePedalStatus_G/1 -> brakeReleventSuppCond/3(BrakePedalStatus_G)
  ESPmasterCylindBrakePress/1 -> brakeReleventSuppCond/2(ESPmasterCylindBrakePress)
  Constant3/1 -> accrlReleventSuppCondStateDelayAbortion/2
  Constant2/1 -> accrlReleventSuppCondStateDelayAbortion/3
  Constant1/1 -> strReleventSuppCondStateDelayAbortion/3
  Constant/1 -> strReleventSuppCondStateDelayAbortion/2
  Logical Operator/1 -> strReleventSuppCondStateDelayAbortion/1
  Logical Operator2/1 -> driverSuppCondFlg/1  "driverSuppCond"
  Compare To Constant4/1 -> accrlReleventSuppCondStateDelayAbortion/1
  accrlReleventSuppCondStateDelayAbortion/1 -> Logical Operator2/3  "accrlReleventSuppCond"
  AccrPedlPosnDiagc/1 -> Compare To Constant4/1
  brakeReleventSuppCond/1(brakeReleventSuppCond) -> Logical Operator2/2  "brakeReleventSuppCond"
  strReleventSuppCondStateDelayAbortion/1 -> Logical Operator2/1  "strReleventSuppCond"
  Compare To Constant1/1 -> Logical Operator/2
  Compare To Constant/1 -> Logical Operator/1
  Abs1/1 -> Compare To Constant1/1
  SteerWheelSpd/1 -> Abs1/1
  Abs/1 -> Compare To Constant/1
  SteerWheelAngle/1 -> Abs/1

== system TosAeb/FcwAebJa_Step_sys/ALG_AEBSystem/ALG_AEBController/AEB_FuncCondCalcApp/LongFunctionCondCalc/vehPrefillSuppAborCondCalc/vehPrefillSuppCondCalc/vehPrefillVehRelActiveCondCalc/subjVelWork ==
  ObjectMovingState : Inport
  Compare To Constant : simulink/Logic and Bit Operations/Compare To Constant  relop===  const=AEB_ObjectMovingState.Still  OutDataTypeStr=boolean
  Constant : Constant  Value=P_AEB_Func_vehPrefillSubjVelMaxActivationThresSO
  Constant1 : Constant  Value=P_AEB_Func_vehPrefillSubjVelMaxActivationThresMO
  Constant2 : Constant  Value=P_AEB_Func_vehPrefillSubjVelMinActivationThres
  Switch : Switch  Criteria=u2 ~= 0
  subjVelWorkMax : Outport
  subjVelWorkMin : Outport  Port=2
  wires:
  Compare To Constant/1 -> Switch/2
  ObjectMovingState/1 -> Compare To Constant/1
  Constant/1 -> Switch/1
  Constant1/1 -> Switch/3
  Switch/1 -> subjVelWorkMax/1
  Constant2/1 -> subjVelWorkMin/1

== system TosAeb/FcwAebJa_Step_sys/ALG_AEBSystem/ALG_AEBController/AEB_FuncCondCalcApp/LongFunctionCondCalc/vehPrefillSuppAborCondCalc/vehPrefillSuppCondCalc/vehPrefillVehRelActiveCondCalc/vehAcuteWarningVehRelActiveCondJudge ==
  subjVel : Inport
  subjVelWorkMax : Inport  Port=2
  subjVelWorkMin : Inport  Port=3
  driverSuppCondFlg : Inport  Port=4
  vehStateCalcPrefillSuppFlg : Inport  Port=5
  Compare To Constant : simulink/Logic and Bit Operations/Compare To Constant  relop===  const=FALSE  OutDataTypeStr=boolean
  Compare To Constant1 : simulink/Logic and Bit Operations/Compare To Constant  relop===  const=FALSE  OutDataTypeStr=boolean
  Logical Operator : Logic  AllPortsSameDT=off  OutDataTypeStr=boolean
  Logical Operator1 : Logic  Inputs=3  AllPortsSameDT=off  OutDataTypeStr=boolean
  Relational Operator : RelationalOperator  Operator=<  OutDataTypeStr=boolean  RndMeth=Simplest
  Relational Operator1 : RelationalOperator  Operator=>  OutDataTypeStr=boolean  RndMeth=Simplest
  vehPrefillVehRelActiveCondFulfilled : Outport
  wires:
  Logical Operator1/1 -> vehPrefillVehRelActiveCondFulfilled/1  "vehPrefillVehRelActiveCondFulfilled"
  Logical Operator/1 -> Logical Operator1/1
  Compare To Constant/1 -> Logical Operator1/2
  vehStateCalcPrefillSuppFlg/1 -> Compare To Constant1/1
  driverSuppCondFlg/1 -> Compare To Constant/1
  Relational Operator1/1 -> Logical Operator/2
  Relational Operator/1 -> Logical Operator/1
  subjVelWorkMin/1 -> Relational Operator1/2
  subjVelWorkMax/1 -> Relational Operator/2
  subjVel/1 -> Relational Operator/1, Relational Operator1/1
  Compare To Constant1/1 -> Logical Operator1/3

== system TosAeb/FcwAebJa_Step_sys/ALG_AEBSystem/ALG_AEBController/AEB_FuncCondCalcApp/PedFunctionCondCalc/pedABASuppAborCond/pedABASuppCondCalc/pedABASituationRelActiveCondCalc/LongDistCond4PreWarningABA ==
  FcwHMISetting : Inport
  subjVel : Inport  Port=2
  dxFrontBumper : Inport  Port=3
  Compare To Constant : simulink/Logic and Bit Operations/Compare To Constant  relop===  const=FCWSnvty.HMISettingEarly  OutDataTypeStr=boolean
  Compare To Constant1 : simulink/Logic and Bit Operations/Compare To Constant  relop===  const=FCWSnvty.HMISettingMiddle  OutDataTypeStr=boolean
  Constant : Constant  Value=P_AEB_Func_pedABALongDistTimeOffsetHmiEarlyMid
  Constant1 : Constant  Value=P_AEB_Func_pedABALongDistTimeOffsetHmiLate
  Logical Operator : Logic  Operator=OR  AllPortsSameDT=off  OutDataTypeStr=boolean
  Product : Product
  Relational Operator : RelationalOperator  Operator=<=  OutDataTypeStr=boolean  RndMeth=Simplest
  Switch : Switch  Criteria=u2 ~= 0
  longDistCond4ABA : Outport
  wires:
  FcwHMISetting/1 -> Compare To Constant1/1, Compare To Constant/1
  Compare To Constant/1 -> Logical Operator/1
  Compare To Constant1/1 -> Logical Operator/2
  Logical Operator/1 -> Switch/2
  Constant/1 -> Switch/1
  Constant1/1 -> Switch/3
  Switch/1 -> Product/1
  subjVel/1 -> Product/2
  Product/1 -> Relational Operator/2
  dxFrontBumper/1 -> Relational Operator/1
  Relational Operator/1 -> longDistCond4ABA/1
